simulink model "Demo_IntelligentTrafficSigns_cs"  (Simulink 7.6)
settings: Solver=FixedStepDiscrete  SolverMode=SingleTasking  StartTime=0.0  StopTime=66  FixedStep=1/10  RelTol=1e-3
model workspace (MAT data in the model file):
  mbox_table : struct (value not decoded)
PreLoadFcn:
  | check_NUTS_defined
  | 
  | assignin('base',['model_closed_' bdroot],false);
InitFcn:
  | PreScanExperimentInitFcn(bdroot)
StopFcn:
  | PreScanExperimentStopFcn(bdroot);
  | 
undefined symbols (not defined in the file or in its folder): DiscIntNeverNeededParam, magnitude, permission, R_curve, Results, Results_20090205_104801, spare_10, spare_4, spare_5, spare_6, spare_7, spare_8, spare_9, velocity_limit, x_ini_VRML, y_ini_VRML, z_ini_VRML
libraries: simulink_extras x8, PreScanSimulinkLibrary x7, simulink x5, PreScanUsefulBlocks x3
(part 1 of 10)
CloseFcn:
  | evalin('base',['clear model_closed_' bdroot]);
settings Model: Creator=PRESCAN  LastModifiedBy=buildbot
settings OptimizationCC: InitFltsAndDblsToZero=on
settings DebuggingCC: SaveWithParameterizedLinksMsg=none  SignalResolutionControl=TryResolveAllWithWarning  MaxConsecutiveZCsMsg=warning  MultiTaskDSMMsg=warning  MultiTaskCondExecSysMsg=none
settings SFSimCC: SimParseCustomCode=off
settings RTWCC: IncludeERTFirstTime=on
settings GRTTargetCC: TargetFcnLib=ansi_tfl_tmw.mat

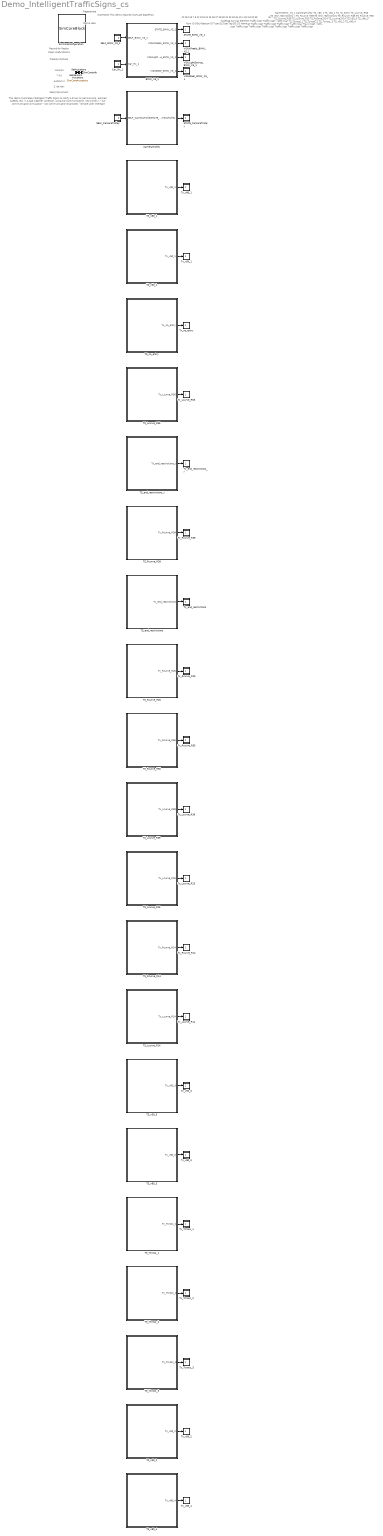
== system Demo_IntelligentTrafficSigns_cs ==
  note: OBU-Beacon ID
  note: Demo Help
  note: WARNING:
      | This demo requires MATLAB StateFlow
  note: Regenerate
  note: Record for Replay
  note: Open Useful Blocks
  note: PreScan Options
  note: Version:
  note: 7.4.0
  note: Author(s):
  note: S. de Hair
  note: Descriptive text:
  note: This demo illustrates Intelligent
      | Traffic Signs to notify a driver
      | on permissions, advised speeds,
      | etc. in a bad weather condition,
      | using C2I communication.
      |
      | Keyword(s):
      | - C2I communication simulation
      | - C2I communication examples
      | - Simple User Interface
  note: ID
      |
      | 28
      | 113
      | 7
      | 6
      | 22
      | 23
      | 8
      | 24
      | 25
      | 26
      | 27
      | 29
      | 30
      | 31
      | 32
      | 33
      | 34
      | 111
      | 112
      | 19
      | 20
      | 38
  note: OBU-Beacon ID
  note: TypeID
      |
      | 1
      | 5
      | 14
      | 14
      | 14
      | 14
      | 14
      | 14
      | 14
      | 14
      | 14
      | 14
      | 14
      | 14
      | 14
      | 14
      | 14
      | 14
      | 14
      | 14
      | 14
      | 14
  note: TypeTag
      |
      | Car
      | Cal element
      | Traffic sign
      | Traffic sign
      | Traffic sign
      | Traffic sign
      | Traffic sign
      | Traffic sign
      | Traffic sign
      | Traffic sign
      | Traffic sign
      | Traffic sign
      | Traffic sign
      | Traffic sign
      | Traffic sign
      | Traffic sign
      | Traffic sign
      | Traffic sign
      | Traffic sign
      | Traffic sign
      | Traffic sign
      | Traffic sign
  note: SD
      |
      | Y
      | Y
      | N
      | N
      | N
      | N
      | N
      | N
      | N
      | N
      | N
      | N
      | N
      | N
      | N
      | N
      | N
      | N
      | N
      | N
      | N
      | N
  note: CD
      |
      | N
      | N
      | N
      | N
      | N
      | N
      | N
      | N
      | N
      | N
      | N
      | N
      | N
      | N
      | N
      | N
      | N
      | N
      | N
      | N
      | N
      | N
  note: Name
      |
      | BMW_X5_1
      | CameraTrolley
      | TS_v80_1
      | TS_v50_1
      | TS_no_entry
      | TS_Lcurve_R55
      | TS_end_restrictions_r
      | TS_Rcurve_R39
      | TS_end_restrictions
      | TS_Rcurve_R25
      | TS_Rcurve_R55
      | TS_Lcurve_R39
      | TS_Lcurve_R25
      | TS_Rcurve_R14
      | TS_Lcurve_R14
      | TS_v50_3
      | TS_v80_3
      | TS_Tcross_1
      | TS_Tcross_2
      | TS_Tcross_3
      | TS_v50_2
      | TS_v80_4
  note: Num ID OBU-Beacon ID Type ID Type Tag SD CD Name
  Actuators : DataStoreMemory  Tag=Actuators  DataStoreName=SimCoreActuators  InitialValue=zeros(4358,1)
      settings: DeleteFcn=PreScanBlockDeleteFcn;
  BMW_X5_1 : Subsystem  Tag=WorldObjectSubSystem  InitFcn=UniqueId = '93';
      mask Display: image(imread('BMW_X5.jpg'));color('white');text(0,0,' ID=28\n')
  CameraTrolley : Subsystem  Tag=WorldObjectSubSystem  InitFcn=UniqueId = '98';
      mask Display: image(imread('Sphere.jpg'));color('white');text(0,0,' ID=113\n')
  Car_Rx_1 : Subsystem  Tag=AntennaR  Priority=3  mask(PreScan Source Block: Antenna Receiver){Channel=1, NrEntitiesOut=5, PortName=Car_Rx_1, PortIndex=8, PortWidth=1140, TimeStep=1/10}
      mask prompts: Channel "User Specified: Channel# [-]"; NrEntitiesOut "User Specified: # of Visible Actors"; PortName "Auto Assigned: Port Name [-]"; PortIndex "Auto Assigned: Index [-]"; PortWidth "Auto Assigned: Port Dimensions [-]"; TimeStep "Auto Assigned: Upload Period [s]"
      mask help:
      | Using the Antenna Receiver (RX) and Transmitter (TX) information between actors can be exchanged. This is done over a channel number to be specified by the user.
      |
      | The Antenna Reciever receives actor information and user-definable signals.
      |
      | For more information, please click Help.
      settings: DeleteFcn=PreScanBlockDeleteFcn;
      mask Help: eval('openHelp(424)')
      mask Display: image(imread('Rx.png'))
  GetSensors : Subsystem  Tag=GetSensors  Priority=2  mask(PreScan Simulation Core Block){NrOfSensors=1156, TimeStep=1/10}
      mask prompts: NrOfSensors "Number of sensor values"; TimeStep "Timestep [s]"
      mask help:
      | Please do not remove this block from the PreScan compilation sheet,
      | as it contains essential configuration parameters for a PreScan simulation.
      settings: DeleteFcn=PreScanBlockDeleteFcn;
  SELF_BMW_X5_1 : Subsystem  Tag=SELF  Priority=3  mask(PreScan Source Block: SELF){PortName=SELF_BMW_X5_1, PortIndex=0, PortWidth=8, TimeStep=1/10}
      mask prompts: PortName "Auto-Assigned: Port Name [-]"; PortIndex "Auto-Assigned: Port Index [-]"; PortWidth "Auto-Assigned: Port Dimensions"; TimeStep "Auto-Assigned: Upload Period [s]"
      mask help:
      | The SELF port shows (state) information of the actor itself. The outputs are position in the experiment's axis system and the GPS coordinates, and the object's absolute velocity and compass heading.
      |
      | For more information, please click Help.
      settings: DeleteFcn=PreScanBlockDeleteFcn;
      mask Help: eval('openHelp(462)')
      mask Display: image(imread('GPS.png'))
  SELF_CameraTrolley : Subsystem  Tag=SELF  Priority=3  mask(PreScan Source Block: SELF){PortName=SELF_CameraTrolley, PortIndex=1148, PortWidth=8, TimeStep=1/10}
      mask prompts: PortName "Auto-Assigned: Port Name [-]"; PortIndex "Auto-Assigned: Port Index [-]"; PortWidth "Auto-Assigned: Port Dimensions"; TimeStep "Auto-Assigned: Upload Period [s]"
      mask help:
      | The SELF port shows (state) information of the actor itself. The outputs are position in the experiment's axis system and the GPS coordinates, and the object's absolute velocity and compass heading.
      |
      | For more information, please click Help.
      settings: DeleteFcn=PreScanBlockDeleteFcn;
      mask Help: eval('openHelp(462)')
      mask Display: image(imread('GPS.png'))
  STATE_BMW_X5_1 : Subsystem  Tag=State  Priority=0  mask(PreScan Sink Block: State){x0=80, y0=1.75, z0=0.69, Roll0=0, Pitch0=0, Yaw0=179.999588, TimeStep=1/10}
      mask prompts: x0 "Ini x-position VEHICLE wrt GLOBAL axis system [m]"; y0 "Ini y-position VEHICLE wrt GLOBAL axis system [m]"; z0 "Ini z-position VEHICLE wrt GLOBAL axis system [m]"; Roll0 "Ini roll-angle BODY wrt VEHICLE axis system [deg]"; Pitch0 "Ini pitch-angle BODY wrt VEHICLE axis system [deg]"; Yaw0 "Ini yaw-angle VEHICLE wrt GLOBAL axis system [deg]"; TimeStep "Time Step [s]"
      mask help:
      | The Actor State contains a vector signal of size (9x1), containing the following signals:
      |
      | -  Position                  (x,y,z)                                     [m]
      | -  Velocity                  (vx,vy,vz)                               [m/s]
      | -  Angles                    (Roll, Pitch, Yaw)                   [rad]
      |
      | The Actor state is defined in the global axis system.
      | The state is superponed on the Initial position defined in the GUI.
      |
      | For more information, please click Help.
      settings: DeleteFcn=PreScanBlockDeleteFcn;
      mask Help: eval('openHelp(222)')
      mask Display: image(imread('State.png'))
  STATE_CameraTrolley : Subsystem  Tag=State  Priority=0  mask(PreScan Sink Block: State){x0=80, y0=1.75, z0=0.69, Roll0=0, Pitch0=0, Yaw0=179.9996, TimeStep=1/10}
      mask prompts: x0 "Ini x-position VEHICLE wrt GLOBAL axis system [m]"; y0 "Ini y-position VEHICLE wrt GLOBAL axis system [m]"; z0 "Ini z-position VEHICLE wrt GLOBAL axis system [m]"; Roll0 "Ini roll-angle BODY wrt VEHICLE axis system [deg]"; Pitch0 "Ini pitch-angle BODY wrt VEHICLE axis system [deg]"; Yaw0 "Ini yaw-angle VEHICLE wrt GLOBAL axis system [deg]"; TimeStep "Time Step [s]"
      mask help:
      | The Actor State contains a vector signal of size (9x1), containing the following signals:
      |
      | -  Position                  (x,y,z)                                     [m]
      | -  Velocity                  (vx,vy,vz)                               [m/s]
      | -  Angles                    (Roll, Pitch, Yaw)                   [rad]
      |
      | The Actor state is defined in the global axis system.
      | The state is superponed on the Initial position defined in the GUI.
      |
      | For more information, please click Help.
      settings: DeleteFcn=PreScanBlockDeleteFcn;
      mask Help: eval('openHelp(222)')
      mask Display: image(imread('State.png'))
  SetActuators : Subsystem  Tag=SetActuators  Priority=1  mask(PreScan Simulation Core Block){TimeStep=1/10, NrOfActuators=4358}
      mask prompts: TimeStep "Timestep [s]"; NrOfActuators "Number of actuator values"
      mask help:
      | Please do not remove this block from the PreScan compilation sheet,
      | as it contains essential configuration parameters for a PreScan simulation.
      settings: DeleteFcn=PreScanBlockDeleteFcn;
  SimCoreConfiguration : M-S-Function  Tag=SimCoreConfiguration  Description=8F06161321348DF6F304B7355CE55985CA9F2FDCA8A28FBBC2716AEAFE3B5E23  FunctionName=SimCoreBlock  Parameters=ExperimentXML PortsXML SimulationCore HostName CheckStart EnableLog RfR EnableGPUAccel ShowComputationTime  mask(PreScan Simulation Core Configurator){SimulationCore=NUTS, HostName=0, CheckStart=0, prescanversion='7.4.0', EnableLog=off, RfR=0, EnableGPUAccel=off, ShowComputationTime=off}
      mask prompts: SimulationCore "Simulation Core"; HostName "Simulation Host"; CheckStart "Show START Dialog"; prescanversion "PreScan Version"; EnableLog "Create TA Log"; RfR "Record for Replay"; EnableGPUAccel "Enable GPU Acceleration"; ShowComputationTime "Show ComputationTime Scope"
      mask help:
      | Please do not remove this block from the PreScan compilation sheet,
      | as it contains essential configuration parameter for a PreScan simulation.
      mask init:
      | model = bdroot;
      | ExperimentXML = [model(1:end-3) '.pex'];
      | PortsXML = [model '_ports.pcd'];
      settings: DeleteFcn=PreScanBlockDeleteFcn;  ModelCloseFcn=assignin('base',['model_closed_' bdroot],true);
      mask Help: eval('openHelp(812)')
      mask Display: image(imread('prescanlogo.ico'))
  SimCoreInfo : Subsystem  Tag=SimCoreInfo
  TS_Lcurve_R14 : Subsystem  Tag=WorldObjectSubSystem  InitFcn=UniqueId = '76';
      mask Display: image(imread('TrafficSignPoleNL.jpg'));color('white');text(0,0,' ID=32\n')
  TS_Lcurve_R25 : Subsystem  Tag=WorldObjectSubSystem  InitFcn=UniqueId = '72';
      mask Display: image(imread('TrafficSignPoleNL.jpg'));color('white');text(0,0,' ID=30\n')
  TS_Lcurve_R39 : Subsystem  Tag=WorldObjectSubSystem  InitFcn=UniqueId = '70';
      mask Display: image(imread('TrafficSignPoleNL.jpg'));color('white');text(0,0,' ID=29\n')
  TS_Lcurve_R55 : Subsystem  Tag=WorldObjectSubSystem  InitFcn=UniqueId = '58';
      mask Display: image(imread('TrafficSignPoleNL.jpg'));color('white');text(0,0,' ID=23\n')
  TS_Rcurve_R14 : Subsystem  Tag=WorldObjectSubSystem  InitFcn=UniqueId = '74';
      mask Display: image(imread('TrafficSignPoleNL.jpg'));color('white');text(0,0,' ID=31\n')
  TS_Rcurve_R25 : Subsystem  Tag=WorldObjectSubSystem  InitFcn=UniqueId = '66';
      mask Display: image(imread('TrafficSignPoleNL.jpg'));color('white');text(0,0,' ID=26\n')
  TS_Rcurve_R39 : Subsystem  Tag=WorldObjectSubSystem  InitFcn=UniqueId = '62';
      mask Display: image(imread('TrafficSignPoleNL.jpg'));color('white');text(0,0,' ID=24\n')
  TS_Rcurve_R55 : Subsystem  Tag=WorldObjectSubSystem  InitFcn=UniqueId = '68';
      mask Display: image(imread('TrafficSignPoleNL.jpg'));color('white');text(0,0,' ID=27\n')
  TS_Tcross_1 : Subsystem  Tag=WorldObjectSubSystem  InitFcn=UniqueId = '82';
      mask Display: image(imread('TrafficSignPoleNL.jpg'));color('white');text(0,0,' ID=111\n')
  TS_Tcross_2 : Subsystem  Tag=WorldObjectSubSystem  InitFcn=UniqueId = '84';
      mask Display: image(imread('TrafficSignPoleNL.jpg'));color('white');text(0,0,' ID=112\n')
  TS_Tcross_3 : Subsystem  Tag=WorldObjectSubSystem  InitFcn=UniqueId = '120';
      mask Display: image(imread('TrafficSignPoleNL.jpg'));color('white');text(0,0,' ID=19\n')
  TS_end_restrictions : Subsystem  Tag=WorldObjectSubSystem  InitFcn=UniqueId = '64';
      mask Display: image(imread('TrafficSignPoleNL.jpg'));color('white');text(0,0,' ID=25\n')
  TS_end_restrictions_r : Subsystem  Tag=WorldObjectSubSystem  InitFcn=UniqueId = '60';
      mask Display: image(imread('TrafficSignPoleNL.jpg'));color('white');text(0,0,' ID=8\n')
  TS_no_entry : Subsystem  Tag=WorldObjectSubSystem  InitFcn=UniqueId = '56';
      mask Display: image(imread('TrafficSignPoleNL.jpg'));color('white');text(0,0,' ID=22\n')
  TS_v50_1 : Subsystem  Tag=WorldObjectSubSystem  InitFcn=UniqueId = '54';
      mask Display: image(imread('TrafficSignPoleNL.jpg'));color('white');text(0,0,' ID=6\n')
  TS_v50_2 : Subsystem  Tag=WorldObjectSubSystem  InitFcn=UniqueId = '122';
      mask Display: image(imread('TrafficSignPoleNL.jpg'));color('white');text(0,0,' ID=20\n')
  TS_v50_3 : Subsystem  Tag=WorldObjectSubSystem  InitFcn=UniqueId = '78';
      mask Display: image(imread('TrafficSignPoleNL.jpg'));color('white');text(0,0,' ID=33\n')
  TS_v80_1 : Subsystem  Tag=WorldObjectSubSystem  InitFcn=UniqueId = '52';
      mask Display: image(imread('TrafficSignPoleNL.jpg'));color('white');text(0,0,' ID=7\n')
  TS_v80_3 : Subsystem  Tag=WorldObjectSubSystem  InitFcn=UniqueId = '80';
      mask Display: image(imread('TrafficSignPoleNL.jpg'));color('white');text(0,0,' ID=34\n')
  TS_v80_4 : Subsystem  Tag=WorldObjectSubSystem  InitFcn=UniqueId = '125';
      mask Display: image(imread('TrafficSignPoleNL.jpg'));color('white');text(0,0,' ID=38\n')
  Tx_Lcurve_R14 : Subsystem  Tag=AntennaT  Priority=0  mask(PreScan Sink Block: AntennaT){Channel=1, PortName=Tx_Lcurve_R14, PortIndex=2718, PortWidth=205, TimeStep=1/10}
      mask prompts: Channel "User Specified: Channel# [-]"; PortName "Auto Assigned: Port Name [-]"; PortIndex "Auto Assigned: Index [-]"; PortWidth "Auto Assigned: Port Dimensions [-]"; TimeStep "Auto Assigned: Upload Period [s]"
      mask help:
      | Using the Antenna Receiver (RX) and Transmitter (TX) information between actors can be exchanged. This is done over a channel number to be specified by the user.
      |
      | The Antenna Transmitter contains the following, user-definable signals:
      |
      | - Warning Signal ID	[-]
      | - Maneuvre ID		[-]
      | - Vehicle Type 		[-]
      | - Braking Capacity	[-]
      | - Vehicle Size		[-]
      | - Spares (1:10)		[-]
      |
      | For more information, please click Help.
      settings: DeleteFcn=PreScanBlockDeleteFcn;
      mask Help: eval('openHelp(424)')
      mask Display: image(imread('Tx.png'))
  Tx_Lcurve_R25 : Subsystem  Tag=AntennaT  Priority=0  mask(PreScan Sink Block: AntennaT){Channel=1, PortName=Tx_Lcurve_R25, PortIndex=2308, PortWidth=205, TimeStep=1/10}
      mask prompts: Channel "User Specified: Channel# [-]"; PortName "Auto Assigned: Port Name [-]"; PortIndex "Auto Assigned: Index [-]"; PortWidth "Auto Assigned: Port Dimensions [-]"; TimeStep "Auto Assigned: Upload Period [s]"
      mask help:
      | Using the Antenna Receiver (RX) and Transmitter (TX) information between actors can be exchanged. This is done over a channel number to be specified by the user.
      |
      | The Antenna Transmitter contains the following, user-definable signals:
      |
      | - Warning Signal ID	[-]
      | - Maneuvre ID		[-]
      | - Vehicle Type 		[-]
      | - Braking Capacity	[-]
      | - Vehicle Size		[-]
      | - Spares (1:10)		[-]
      |
      | For more information, please click Help.
      settings: DeleteFcn=PreScanBlockDeleteFcn;
      mask Help: eval('openHelp(424)')
      mask Display: image(imread('Tx.png'))
  Tx_Lcurve_R39 : Subsystem  Tag=AntennaT  Priority=0  mask(PreScan Sink Block: AntennaT){Channel=1, PortName=Tx_Lcurve_R39, PortIndex=2103, PortWidth=205, TimeStep=1/10}
      mask prompts: Channel "User Specified: Channel# [-]"; PortName "Auto Assigned: Port Name [-]"; PortIndex "Auto Assigned: Index [-]"; PortWidth "Auto Assigned: Port Dimensions [-]"; TimeStep "Auto Assigned: Upload Period [s]"
      mask help:
      | Using the Antenna Receiver (RX) and Transmitter (TX) information between actors can be exchanged. This is done over a channel number to be specified by the user.
      |
      | The Antenna Transmitter contains the following, user-definable signals:
      |
      | - Warning Signal ID	[-]
      | - Maneuvre ID		[-]
      | - Vehicle Type 		[-]
      | - Braking Capacity	[-]
      | - Vehicle Size		[-]
      | - Spares (1:10)		[-]
      |
      | For more information, please click Help.
      settings: DeleteFcn=PreScanBlockDeleteFcn;
      mask Help: eval('openHelp(424)')
      mask Display: image(imread('Tx.png'))
  Tx_Lcurve_R55 : Subsystem  Tag=AntennaT  Priority=0  mask(PreScan Sink Block: AntennaT){Channel=1, PortName=Tx_Lcurve_R55, PortIndex=873, PortWidth=205, TimeStep=1/10}
      mask prompts: Channel "User Specified: Channel# [-]"; PortName "Auto Assigned: Port Name [-]"; PortIndex "Auto Assigned: Index [-]"; PortWidth "Auto Assigned: Port Dimensions [-]"; TimeStep "Auto Assigned: Upload Period [s]"
      mask help:
      | Using the Antenna Receiver (RX) and Transmitter (TX) information between actors can be exchanged. This is done over a channel number to be specified by the user.
      |
      | The Antenna Transmitter contains the following, user-definable signals:
      |
      | - Warning Signal ID	[-]
      | - Maneuvre ID		[-]
      | - Vehicle Type 		[-]
      | - Braking Capacity	[-]
      | - Vehicle Size		[-]
      | - Spares (1:10)		[-]
      |
      | For more information, please click Help.
      settings: DeleteFcn=PreScanBlockDeleteFcn;
      mask Help: eval('openHelp(424)')
      mask Display: image(imread('Tx.png'))
  Tx_Rcurve_R14 : Subsystem  Tag=AntennaT  Priority=0  mask(PreScan Sink Block: AntennaT){Channel=1, PortName=Tx_Rcurve_R14, PortIndex=2513, PortWidth=205, TimeStep=1/10}
      mask prompts: Channel "User Specified: Channel# [-]"; PortName "Auto Assigned: Port Name [-]"; PortIndex "Auto Assigned: Index [-]"; PortWidth "Auto Assigned: Port Dimensions [-]"; TimeStep "Auto Assigned: Upload Period [s]"
      mask help:
      | Using the Antenna Receiver (RX) and Transmitter (TX) information between actors can be exchanged. This is done over a channel number to be specified by the user.
      |
      | The Antenna Transmitter contains the following, user-definable signals:
      |
      | - Warning Signal ID	[-]
      | - Maneuvre ID		[-]
      | - Vehicle Type 		[-]
      | - Braking Capacity	[-]
      | - Vehicle Size		[-]
      | - Spares (1:10)		[-]
      |
      | For more information, please click Help.
      settings: DeleteFcn=PreScanBlockDeleteFcn;
      mask Help: eval('openHelp(424)')
      mask Display: image(imread('Tx.png'))
  Tx_Rcurve_R25 : Subsystem  Tag=AntennaT  Priority=0  mask(PreScan Sink Block: AntennaT){Channel=1, PortName=Tx_Rcurve_R25, PortIndex=1693, PortWidth=205, TimeStep=1/10}
      mask prompts: Channel "User Specified: Channel# [-]"; PortName "Auto Assigned: Port Name [-]"; PortIndex "Auto Assigned: Index [-]"; PortWidth "Auto Assigned: Port Dimensions [-]"; TimeStep "Auto Assigned: Upload Period [s]"
      mask help:
      | Using the Antenna Receiver (RX) and Transmitter (TX) information between actors can be exchanged. This is done over a channel number to be specified by the user.
      |
      | The Antenna Transmitter contains the following, user-definable signals:
      |
      | - Warning Signal ID	[-]
      | - Maneuvre ID		[-]
      | - Vehicle Type 		[-]
      | - Braking Capacity	[-]
      | - Vehicle Size		[-]
      | - Spares (1:10)		[-]
      |
      | For more information, please click Help.
      settings: DeleteFcn=PreScanBlockDeleteFcn;
      mask Help: eval('openHelp(424)')
      mask Display: image(imread('Tx.png'))
  Tx_Rcurve_R39 : Subsystem  Tag=AntennaT  Priority=0  mask(PreScan Sink Block: AntennaT){Channel=1, PortName=Tx_Rcurve_R39, PortIndex=1283, PortWidth=205, TimeStep=1/10}
      mask prompts: Channel "User Specified: Channel# [-]"; PortName "Auto Assigned: Port Name [-]"; PortIndex "Auto Assigned: Index [-]"; PortWidth "Auto Assigned: Port Dimensions [-]"; TimeStep "Auto Assigned: Upload Period [s]"
      mask help:
      | Using the Antenna Receiver (RX) and Transmitter (TX) information between actors can be exchanged. This is done over a channel number to be specified by the user.
      |
      | The Antenna Transmitter contains the following, user-definable signals:
      |
      | - Warning Signal ID	[-]
      | - Maneuvre ID		[-]
      | - Vehicle Type 		[-]
      | - Braking Capacity	[-]
      | - Vehicle Size		[-]
      | - Spares (1:10)		[-]
      |
      | For more information, please click Help.
      settings: DeleteFcn=PreScanBlockDeleteFcn;
      mask Help: eval('openHelp(424)')
      mask Display: image(imread('Tx.png'))
  Tx_Rcurve_R55 : Subsystem  Tag=AntennaT  Priority=0  mask(PreScan Sink Block: AntennaT){Channel=1, PortName=Tx_Rcurve_R55, PortIndex=1898, PortWidth=205, TimeStep=1/10}
      mask prompts: Channel "User Specified: Channel# [-]"; PortName "Auto Assigned: Port Name [-]"; PortIndex "Auto Assigned: Index [-]"; PortWidth "Auto Assigned: Port Dimensions [-]"; TimeStep "Auto Assigned: Upload Period [s]"
      mask help:
      | Using the Antenna Receiver (RX) and Transmitter (TX) information between actors can be exchanged. This is done over a channel number to be specified by the user.
      |
      | The Antenna Transmitter contains the following, user-definable signals:
      |
      | - Warning Signal ID	[-]
      | - Maneuvre ID		[-]
      | - Vehicle Type 		[-]
      | - Braking Capacity	[-]
      | - Vehicle Size		[-]
      | - Spares (1:10)		[-]
      |
      | For more information, please click Help.
      settings: DeleteFcn=PreScanBlockDeleteFcn;
      mask Help: eval('openHelp(424)')
      mask Display: image(imread('Tx.png'))
  Tx_Tcross_1 : Subsystem  Tag=AntennaT  Priority=0  mask(PreScan Sink Block: AntennaT){Channel=1, PortName=Tx_Tcross_1, PortIndex=3333, PortWidth=205, TimeStep=1/10}
      mask prompts: Channel "User Specified: Channel# [-]"; PortName "Auto Assigned: Port Name [-]"; PortIndex "Auto Assigned: Index [-]"; PortWidth "Auto Assigned: Port Dimensions [-]"; TimeStep "Auto Assigned: Upload Period [s]"
      mask help:
      | Using the Antenna Receiver (RX) and Transmitter (TX) information between actors can be exchanged. This is done over a channel number to be specified by the user.
      |
      | The Antenna Transmitter contains the following, user-definable signals:
      |
      | - Warning Signal ID	[-]
      | - Maneuvre ID		[-]
      | - Vehicle Type 		[-]
      | - Braking Capacity	[-]
      | - Vehicle Size		[-]
      | - Spares (1:10)		[-]
      |
      | For more information, please click Help.
      settings: DeleteFcn=PreScanBlockDeleteFcn;
      mask Help: eval('openHelp(424)')
      mask Display: image(imread('Tx.png'))
  Tx_Tcross_2 : Subsystem  Tag=AntennaT  Priority=0  mask(PreScan Sink Block: AntennaT){Channel=1, PortName=Tx_Tcross_2, PortIndex=3538, PortWidth=205, TimeStep=1/10}
      mask prompts: Channel "User Specified: Channel# [-]"; PortName "Auto Assigned: Port Name [-]"; PortIndex "Auto Assigned: Index [-]"; PortWidth "Auto Assigned: Port Dimensions [-]"; TimeStep "Auto Assigned: Upload Period [s]"
      mask help:
      | Using the Antenna Receiver (RX) and Transmitter (TX) information between actors can be exchanged. This is done over a channel number to be specified by the user.
      |
      | The Antenna Transmitter contains the following, user-definable signals:
      |
      | - Warning Signal ID	[-]
      | - Maneuvre ID		[-]
      | - Vehicle Type 		[-]
      | - Braking Capacity	[-]
      | - Vehicle Size		[-]
      | - Spares (1:10)		[-]
      |
      | For more information, please click Help.
      settings: DeleteFcn=PreScanBlockDeleteFcn;
      mask Help: eval('openHelp(424)')
      mask Display: image(imread('Tx.png'))
  Tx_Tcross_3 : Subsystem  Tag=AntennaT  Priority=0  mask(PreScan Sink Block: AntennaT){Channel=1, PortName=Tx_Tcross_3, PortIndex=3743, PortWidth=205, TimeStep=1/10}
      mask prompts: Channel "User Specified: Channel# [-]"; PortName "Auto Assigned: Port Name [-]"; PortIndex "Auto Assigned: Index [-]"; PortWidth "Auto Assigned: Port Dimensions [-]"; TimeStep "Auto Assigned: Upload Period [s]"
      mask help:
      | Using the Antenna Receiver (RX) and Transmitter (TX) information between actors can be exchanged. This is done over a channel number to be specified by the user.
      |
      | The Antenna Transmitter contains the following, user-definable signals:
      |
      | - Warning Signal ID	[-]
      | - Maneuvre ID		[-]
      | - Vehicle Type 		[-]
      | - Braking Capacity	[-]
      | - Vehicle Size		[-]
      | - Spares (1:10)		[-]
      |
      | For more information, please click Help.
      settings: DeleteFcn=PreScanBlockDeleteFcn;
      mask Help: eval('openHelp(424)')
      mask Display: image(imread('Tx.png'))
  Tx_end_restrictions : Subsystem  Tag=AntennaT  Priority=0  mask(PreScan Sink Block: AntennaT){Channel=1, PortName=Tx_end_restrictions, PortIndex=1488, PortWidth=205, TimeStep=1/10}
      mask prompts: Channel "User Specified: Channel# [-]"; PortName "Auto Assigned: Port Name [-]"; PortIndex "Auto Assigned: Index [-]"; PortWidth "Auto Assigned: Port Dimensions [-]"; TimeStep "Auto Assigned: Upload Period [s]"
      mask help:
      | Using the Antenna Receiver (RX) and Transmitter (TX) information between actors can be exchanged. This is done over a channel number to be specified by the user.
      |
      | The Antenna Transmitter contains the following, user-definable signals:
      |
      | - Warning Signal ID	[-]
      | - Maneuvre ID		[-]
      | - Vehicle Type 		[-]
      | - Braking Capacity	[-]
      | - Vehicle Size		[-]
      | - Spares (1:10)		[-]
      |
      | For more information, please click Help.
      settings: DeleteFcn=PreScanBlockDeleteFcn;
      mask Help: eval('openHelp(424)')
      mask Display: image(imread('Tx.png'))
  Tx_end_restrictions_r : Subsystem  Tag=AntennaT  Priority=0  mask(PreScan Sink Block: AntennaT){Channel=1, PortName=Tx_end_restrictions_r, PortIndex=1078, PortWidth=205, TimeStep=1/10}
      mask prompts: Channel "User Specified: Channel# [-]"; PortName "Auto Assigned: Port Name [-]"; PortIndex "Auto Assigned: Index [-]"; PortWidth "Auto Assigned: Port Dimensions [-]"; TimeStep "Auto Assigned: Upload Period [s]"
      mask help:
      | Using the Antenna Receiver (RX) and Transmitter (TX) information between actors can be exchanged. This is done over a channel number to be specified by the user.
      |
      | The Antenna Transmitter contains the following, user-definable signals:
      |
      | - Warning Signal ID	[-]
      | - Maneuvre ID		[-]
      | - Vehicle Type 		[-]
      | - Braking Capacity	[-]
      | - Vehicle Size		[-]
      | - Spares (1:10)		[-]
      |
      | For more information, please click Help.
      settings: DeleteFcn=PreScanBlockDeleteFcn;
      mask Help: eval('openHelp(424)')
      mask Display: image(imread('Tx.png'))
  Tx_no_entry : Subsystem  Tag=AntennaT  Priority=0  mask(PreScan Sink Block: AntennaT){Channel=1, PortName=Tx_no_entry, PortIndex=668, PortWidth=205, TimeStep=1/10}
      mask prompts: Channel "User Specified: Channel# [-]"; PortName "Auto Assigned: Port Name [-]"; PortIndex "Auto Assigned: Index [-]"; PortWidth "Auto Assigned: Port Dimensions [-]"; TimeStep "Auto Assigned: Upload Period [s]"
      mask help:
      | Using the Antenna Receiver (RX) and Transmitter (TX) information between actors can be exchanged. This is done over a channel number to be specified by the user.
      |
      | The Antenna Transmitter contains the following, user-definable signals:
      |
      | - Warning Signal ID	[-]
      | - Maneuvre ID		[-]
      | - Vehicle Type 		[-]
      | - Braking Capacity	[-]
      | - Vehicle Size		[-]
      | - Spares (1:10)		[-]
      |
      | For more information, please click Help.
      settings: DeleteFcn=PreScanBlockDeleteFcn;
      mask Help: eval('openHelp(424)')
      mask Display: image(imread('Tx.png'))
  Tx_v50_1 : Subsystem  Tag=AntennaT  Priority=0  mask(PreScan Sink Block: AntennaT){Channel=1, PortName=Tx_v50_1, PortIndex=463, PortWidth=205, TimeStep=1/10}
      mask prompts: Channel "User Specified: Channel# [-]"; PortName "Auto Assigned: Port Name [-]"; PortIndex "Auto Assigned: Index [-]"; PortWidth "Auto Assigned: Port Dimensions [-]"; TimeStep "Auto Assigned: Upload Period [s]"
      mask help:
      | Using the Antenna Receiver (RX) and Transmitter (TX) information between actors can be exchanged. This is done over a channel number to be specified by the user.
      |
      | The Antenna Transmitter contains the following, user-definable signals:
      |
      | - Warning Signal ID	[-]
      | - Maneuvre ID		[-]
      | - Vehicle Type 		[-]
      | - Braking Capacity	[-]
      | - Vehicle Size		[-]
      | - Spares (1:10)		[-]
      |
      | For more information, please click Help.
      settings: DeleteFcn=PreScanBlockDeleteFcn;
      mask Help: eval('openHelp(424)')
      mask Display: image(imread('Tx.png'))
  Tx_v50_2 : Subsystem  Tag=AntennaT  Priority=0  mask(PreScan Sink Block: AntennaT){Channel=1, PortName=Tx_v50_2, PortIndex=3948, PortWidth=205, TimeStep=1/10}
      mask prompts: Channel "User Specified: Channel# [-]"; PortName "Auto Assigned: Port Name [-]"; PortIndex "Auto Assigned: Index [-]"; PortWidth "Auto Assigned: Port Dimensions [-]"; TimeStep "Auto Assigned: Upload Period [s]"
      mask help:
      | Using the Antenna Receiver (RX) and Transmitter (TX) information between actors can be exchanged. This is done over a channel number to be specified by the user.
      |
      | The Antenna Transmitter contains the following, user-definable signals:
      |
      | - Warning Signal ID	[-]
      | - Maneuvre ID		[-]
      | - Vehicle Type 		[-]
      | - Braking Capacity	[-]
      | - Vehicle Size		[-]
      | - Spares (1:10)		[-]
      |
      | For more information, please click Help.
      settings: DeleteFcn=PreScanBlockDeleteFcn;
      mask Help: eval('openHelp(424)')
      mask Display: image(imread('Tx.png'))
  Tx_v50_3 : Subsystem  Tag=AntennaT  Priority=0  mask(PreScan Sink Block: AntennaT){Channel=1, PortName=Tx_v50_3, PortIndex=2923, PortWidth=205, TimeStep=1/10}
      mask prompts: Channel "User Specified: Channel# [-]"; PortName "Auto Assigned: Port Name [-]"; PortIndex "Auto Assigned: Index [-]"; PortWidth "Auto Assigned: Port Dimensions [-]"; TimeStep "Auto Assigned: Upload Period [s]"
      mask help:
      | Using the Antenna Receiver (RX) and Transmitter (TX) information between actors can be exchanged. This is done over a channel number to be specified by the user.
      |
      | The Antenna Transmitter contains the following, user-definable signals:
      |
      | - Warning Signal ID	[-]
      | - Maneuvre ID		[-]
      | - Vehicle Type 		[-]
      | - Braking Capacity	[-]
      | - Vehicle Size		[-]
      | - Spares (1:10)		[-]
      |
      | For more information, please click Help.
      settings: DeleteFcn=PreScanBlockDeleteFcn;
      mask Help: eval('openHelp(424)')
      mask Display: image(imread('Tx.png'))
  Tx_v80_1 : Subsystem  Tag=AntennaT  Priority=0  mask(PreScan Sink Block: AntennaT){Channel=1, PortName=Tx_v80_1, PortIndex=258, PortWidth=205, TimeStep=1/10}
      mask prompts: Channel "User Specified: Channel# [-]"; PortName "Auto Assigned: Port Name [-]"; PortIndex "Auto Assigned: Index [-]"; PortWidth "Auto Assigned: Port Dimensions [-]"; TimeStep "Auto Assigned: Upload Period [s]"
      mask help:
      | Using the Antenna Receiver (RX) and Transmitter (TX) information between actors can be exchanged. This is done over a channel number to be specified by the user.
      |
      | The Antenna Transmitter contains the following, user-definable signals:
      |
      | - Warning Signal ID	[-]
      | - Maneuvre ID		[-]
      | - Vehicle Type 		[-]
      | - Braking Capacity	[-]
      | - Vehicle Size		[-]
      | - Spares (1:10)		[-]
      |
      | For more information, please click Help.
      settings: DeleteFcn=PreScanBlockDeleteFcn;
      mask Help: eval('openHelp(424)')
      mask Display: image(imread('Tx.png'))
  Tx_v80_3 : Subsystem  Tag=AntennaT  Priority=0  mask(PreScan Sink Block: AntennaT){Channel=1, PortName=Tx_v80_3, PortIndex=3128, PortWidth=205, TimeStep=1/10}
      mask prompts: Channel "User Specified: Channel# [-]"; PortName "Auto Assigned: Port Name [-]"; PortIndex "Auto Assigned: Index [-]"; PortWidth "Auto Assigned: Port Dimensions [-]"; TimeStep "Auto Assigned: Upload Period [s]"
      mask help:
      | Using the Antenna Receiver (RX) and Transmitter (TX) information between actors can be exchanged. This is done over a channel number to be specified by the user.
      |
      | The Antenna Transmitter contains the following, user-definable signals:
      |
      | - Warning Signal ID	[-]
      | - Maneuvre ID		[-]
      | - Vehicle Type 		[-]
      | - Braking Capacity	[-]
      | - Vehicle Size		[-]
      | - Spares (1:10)		[-]
      |
      | For more information, please click Help.
      settings: DeleteFcn=PreScanBlockDeleteFcn;
      mask Help: eval('openHelp(424)')
      mask Display: image(imread('Tx.png'))
  Tx_v80_4 : Subsystem  Tag=AntennaT  Priority=0  mask(PreScan Sink Block: AntennaT){Channel=1, PortName=Tx_v80_4, PortIndex=4153, PortWidth=205, TimeStep=1/10}
      mask prompts: Channel "User Specified: Channel# [-]"; PortName "Auto Assigned: Port Name [-]"; PortIndex "Auto Assigned: Index [-]"; PortWidth "Auto Assigned: Port Dimensions [-]"; TimeStep "Auto Assigned: Upload Period [s]"
      mask help:
      | Using the Antenna Receiver (RX) and Transmitter (TX) information between actors can be exchanged. This is done over a channel number to be specified by the user.
      |
      | The Antenna Transmitter contains the following, user-definable signals:
      |
      | - Warning Signal ID	[-]
      | - Maneuvre ID		[-]
      | - Vehicle Type 		[-]
      | - Braking Capacity	[-]
      | - Vehicle Size		[-]
      | - Spares (1:10)		[-]
      |
      | For more information, please click Help.
      settings: DeleteFcn=PreScanBlockDeleteFcn;
      mask Help: eval('openHelp(424)')
      mask Display: image(imread('Tx.png'))
  VISULightSource_BMW_X5_1 : Subsystem  Tag=VisuLightSource  Priority=0
      settings: DeleteFcn=PreScanBlockDeleteFcn;
      mask Type: PreScan Sink Block: VisuLightSource
      mask Description:
      | The VisuLightSource block provides a way to animate Light Sources of the indicator, braking, fog and main lights of a vehicle.
      | 
      | For more information, please click Help.
      mask Help: eval('openHelp(223)')
      mask Display: image(imread('RearLight.png'))
  VISUSteer_BMW_X5_1 : Subsystem  Tag=VisuSteer  Priority=0
      settings: DeleteFcn=PreScanBlockDeleteFcn;
      mask Type: PreScan Sink Block: VisuSteer
      mask Description:
      | The VisuSteer block provides a way to adapt the orientation of the steering column and steering wheel of a vehicle.
      | 
      | For more information, please click Help.
      mask Help: eval('openHelp(223)')
      mask Display: image(imread('steeringwheel.png'))
  VISUWheels_BMW_X5_1 : Subsystem  Tag=VisuWheels  Priority=0
      settings: DeleteFcn=PreScanBlockDeleteFcn;
      mask Type: PreScan Sink Block: VisuWheels
      mask Description:
      | The VisuWheels block provides a way to animate the wheels of a vehicle, with steering, rotation (because of velocity) and z-displacement.
      | 
      | For more information, please click Help.
      mask Help: eval('openHelp(223)')
      mask Display: image(imread('wheel.png'))
  wires:
  BMW_X5_1/1(STATE_BMW_X5_1) -> STATE_BMW_X5_1/1(State)
  SELF_BMW_X5_1/1(U) -> BMW_X5_1/1(SELF_BMW_X5_1)
  Car_Rx_1/1(U) -> BMW_X5_1/2(Car_Rx_1)
  BMW_X5_1/2(VISUWheels_BMW_X5_1) -> VISUWheels_BMW_X5_1/1(Wheels)
  BMW_X5_1/3(VISULightSource_BMW_X5_1) -> VISULightSource_BMW_X5_1/1(Lights)
  BMW_X5_1/4(VISUSteer_BMW_X5_1) -> VISUSteer_BMW_X5_1/1(Steer)
  CameraTrolley/1(STATE_CameraTrolley) -> STATE_CameraTrolley/1(State)
  SELF_CameraTrolley/1(U) -> CameraTrolley/1(SELF_CameraTrolley)
  TS_v80_1/1(Tx_v80_1) -> Tx_v80_1/1(Y)
  TS_v50_1/1(Tx_v50_1) -> Tx_v50_1/1(Y)
  TS_no_entry/1(Tx_no_entry) -> Tx_no_entry/1(Y)
  TS_Lcurve_R55/1(Tx_Lcurve_R55) -> Tx_Lcurve_R55/1(Y)
  TS_end_restrictions_r/1(Tx_end_restrictions_r) -> Tx_end_restrictions_r/1(Y)
  TS_Rcurve_R39/1(Tx_Rcurve_R39) -> Tx_Rcurve_R39/1(Y)
  TS_end_restrictions/1(Tx_end_restrictions) -> Tx_end_restrictions/1(Y)
  TS_Rcurve_R25/1(Tx_Rcurve_R25) -> Tx_Rcurve_R25/1(Y)
  TS_Rcurve_R55/1(Tx_Rcurve_R55) -> Tx_Rcurve_R55/1(Y)
  TS_Lcurve_R39/1(Tx_Lcurve_R39) -> Tx_Lcurve_R39/1(Y)
  TS_Lcurve_R25/1(Tx_Lcurve_R25) -> Tx_Lcurve_R25/1(Y)
  TS_Rcurve_R14/1(Tx_Rcurve_R14) -> Tx_Rcurve_R14/1(Y)
  TS_Lcurve_R14/1(Tx_Lcurve_R14) -> Tx_Lcurve_R14/1(Y)
  TS_v50_3/1(Tx_v50_3) -> Tx_v50_3/1(Y)
  TS_v80_3/1(Tx_v80_3) -> Tx_v80_3/1(Y)
  TS_Tcross_1/1(Tx_Tcross_1) -> Tx_Tcross_1/1(Y)
  TS_Tcross_2/1(Tx_Tcross_2) -> Tx_Tcross_2/1(Y)
  TS_Tcross_3/1(Tx_Tcross_3) -> Tx_Tcross_3/1(Y)
  TS_v50_2/1(Tx_v50_2) -> Tx_v50_2/1(Y)
  TS_v80_4/1(Tx_v80_4) -> Tx_v80_4/1(Y)
  SetActuators/1(dummy) -> GetSensors/1(dummy)

== system Demo_IntelligentTrafficSigns_cs/BMW_X5_1 ==
  SELF_BMW_X5_1 : Inport  Tag=SELFInport
  Car_Rx_1 : Inport  Tag=AntennaRInport  Port=2
  ANIMATION PORTS Tuning : Subsystem
  Car_Rx_1_BusSelector : BusSelector  Tag=BusAntennaR  OutputSignals=velocity_limit,permission,R_curve
  Car_Rx_1_Demux : Subsystem  Tag=DemuxAntennaR  mask(PreScan Demux Block:){Channel=1}
      settings: DeleteFcn=PreScanBlockDeleteFcn;
      mask Display:
      | fprintf('Channel: %d',Channel)
      | port_label('output', 1, 'Vehicle_ID')
      | port_label('output', 2, 'GPS Pos Lat [deg]')
      | port_label('output', 3, 'GPS Pos Long [deg]')
      | port_label('output', 4, 'GPS Pos Alt [m]')
      | port_label('output', 5, 'Velocity [m/s]')
      | port_label('output', 6, 'Acceleration [m/s2]')
      | port_label('output', 7, 'Heading [deg]')
      | port_label('output', 8, 'Warning signal ID')
      | port_label('output', 9, 'Maneuvre ID')
      | port_label('output', 10, 'Vehicle Type')
      | port_label('output', 11, 'Braking Capacity')
      | port_label('output', 12, 'Vehicle Size')
      | port_label('output', 13, 'Spare')
  Ground_{1..10,13} (11 alike) : Ground
  INTERPRETATION ALGORITHM TrafficSign Intelligence : Subsystem
      mask Display: image(imread('Pictures\TS_detection_algorithm.jpg'))
  SELF_Demux : Subsystem  Tag=DemuxSELF
      settings: DeleteFcn=PreScanBlockDeleteFcn;
      mask Type: PreScan Demux Block:
      mask Display:
      | port_label('output',1,'x [m]')
      | port_label('output',2,'y [m]')
      | port_label('output',3,'z [m]','texmode','on')
      | port_label('output',4,'Rotx [deg]','texmode','on')
      | port_label('output',5,'Roty [deg]','texmode','on')
      | port_label('output',6,'Rotz [deg]','texmode','on')
      | port_label('output',7,'GPS lat [deg]','texmode','on')
      | port_label('output',8,'GPS long [deg]','texmode','on')
      | port_label('output',9,'GPS alt [m]','texmode','on')
      | port_label('output',10,'Velocity [m/s]')
      | port_label('output',11,'Heading [deg]')
      | port_label('output',12,'Yaw rate [deg/s]')
  Terminator_{1..15} (15 alike) : Terminator
  Trajectories : Subsystem  Tag=Trajectories
      mask Display: image(imread('Trajectory.png'));
  VISULightSource_Mux : Subsystem  Tag=MuxLightSource
      settings: DeleteFcn=PreScanBlockDeleteFcn;
      mask Type: PreScan Mux Block:
  VISUSteer_Mux : Subsystem  Tag=MuxSteer
      settings: DeleteFcn=PreScanBlockDeleteFcn;
      mask Type: PreScan Mux Block:
  VISUWheels_Mux : Subsystem  Tag=MuxWheels  mask(PreScan Mux Block:){TimeStep=1/10}
      mask prompts: TimeStep "Auto Assigned: Time Step [s]"
      settings: DeleteFcn=PreScanBlockDeleteFcn;
  STATE_BMW_X5_1 : Outport  Tag=StateOutport
  VISUWheels_BMW_X5_1 : Outport  Tag=VisuWheelsOutport  Port=2  SampleTime=1/10
  VISULightSource_BMW_X5_1 : Outport  Tag=VisuLightsOutport  Port=3  SampleTime=1/10
  VISUSteer_BMW_X5_1 : Outport  Tag=VisuSteerOutport  Port=4  SampleTime=1/10
  tag scope: State_BMW=global
  wires:
  INTERPRETATION ALGORITHM TrafficSign Intelligence/1(delta_v [m/s]) -> [delta_v]
  [State_BMW] -> ANIMATION PORTS Tuning/2(state)
  [delta_v] -> ANIMATION PORTS Tuning/1(v_corr)
  SELF_BMW_X5_1/1 -> SELF_Demux/1(SELF)
  Car_Rx_1_Demux/13(spare) -> Car_Rx_1_BusSelector/1
  Car_Rx_1/1 -> Car_Rx_1_Demux/1(AntennaR)
  VISUWheels_Mux/1(Wheels) -> VISUWheels_BMW_X5_1/1
  VISULightSource_Mux/1(Lights) -> VISULightSource_BMW_X5_1/1
  VISUSteer_Mux/1(Steer) -> VISUSteer_BMW_X5_1/1
  Trajectories/1(State) -> STATE_BMW_X5_1/1, [State_BMW]
  ANIMATION PORTS Tuning/7(Brake [-]) -> VISULightSource_Mux/11(BrakeLightLeft), VISULightSource_Mux/12(BrakeLightRight)
  Ground_13/1 -> VISULightSource_Mux/3(FogLightRearLeft)
  [delta_v] -> Trajectories/1(v_corr [m/s])
  Car_Rx_1_BusSelector/1 -> INTERPRETATION ALGORITHM TrafficSign Intelligence/10(velocity_limits [km/h])  "<velocity_limit>"
  ANIMATION PORTS Tuning/6(zDisp RR [m]) -> VISUWheels_Mux/7(zDisp RR [m])
  ANIMATION PORTS Tuning/4(zDisp FR [m]) -> VISUWheels_Mux/5(zDisp FR [m])
  ANIMATION PORTS Tuning/2(Velocity [m/s]) -> VISUWheels_Mux/3(Velocity [m/s])
  ANIMATION PORTS Tuning/5(zDisp RL [m]) -> VISUWheels_Mux/6(zDisp RL [m])
  ANIMATION PORTS Tuning/3(zDisp FL [m]) -> VISUWheels_Mux/4(zDisp FL [m])
  ANIMATION PORTS Tuning/1(Steer Angle Front [deg]) -> VISUWheels_Mux/1(Steer Angle Front [deg])
  ANIMATION PORTS Tuning/9(Steer [deg]) -> VISUSteer_Mux/2(Steer [deg])
  ANIMATION PORTS Tuning/8(Column [deg]) -> VISUSteer_Mux/1(Column [deg])
  Car_Rx_1_Demux/3(GPS_pos_long_[deg]) -> INTERPRETATION ALGORITHM TrafficSign Intelligence/7(longs [deg])
  Car_Rx_1_Demux/2(GPS_pos_lat_[deg]) -> INTERPRETATION ALGORITHM TrafficSign Intelligence/6(lats [deg])
  Car_Rx_1_Demux/1(Vehicle_ID) -> INTERPRETATION ALGORITHM TrafficSign Intelligence/5(ids [-])
  SELF_Demux/11(heading [deg]) -> INTERPRETATION ALGORITHM TrafficSign Intelligence/4(heading-own [deg])
  SELF_Demux/10(velocity [m/s]) -> INTERPRETATION ALGORITHM TrafficSign Intelligence/3(v_host [m/s])
  SELF_Demux/2(y [m]) -> INTERPRETATION ALGORITHM TrafficSign Intelligence/2(y_host [m])
  SELF_Demux/1(x [m]) -> INTERPRETATION ALGORITHM TrafficSign Intelligence/1(x_host [m])
  Car_Rx_1_Demux/4(GPS_pos_alt_[m]) -> INTERPRETATION ALGORITHM TrafficSign Intelligence/8(alts [m])
  Car_Rx_1_Demux/7(Heading [deg]) -> INTERPRETATION ALGORITHM TrafficSign Intelligence/9(headings [deg])
  Car_Rx_1_BusSelector/3 -> INTERPRETATION ALGORITHM TrafficSign Intelligence/12(R_curves [m])  "<R_curve>"
  Car_Rx_1_BusSelector/2 -> INTERPRETATION ALGORITHM TrafficSign Intelligence/11(notifications [-])  "<permission>"
  Car_Rx_1_Demux/5(Velocity_[m/s]) -> Terminator_1/1
  Car_Rx_1_Demux/6(Acceleration_[m/s2]) -> Terminator_2/1
  Car_Rx_1_Demux/8(warning_signal_id) -> Terminator_3/1
  Car_Rx_1_Demux/9(maneuvre_id) -> Terminator_4/1
  Car_Rx_1_Demux/10(vehicle_type) -> Terminator_5/1
  Car_Rx_1_Demux/11(brakingcapacity) -> Terminator_6/1
  Car_Rx_1_Demux/12(vehicle_size) -> Terminator_7/1
  SELF_Demux/3(z [m]) -> Terminator_8/1
  SELF_Demux/4(rotx [deg]) -> Terminator_9/1
  SELF_Demux/5(roty [deg]) -> Terminator_10/1
  SELF_Demux/6(rotz [deg]) -> Terminator_11/1
  SELF_Demux/7(GPS lat [deg]) -> Terminator_12/1
  SELF_Demux/8(GPS long [deg]) -> Terminator_13/1
  SELF_Demux/9(GPS alt [m]) -> Terminator_14/1
  SELF_Demux/12(yaw rate [deg/s]) -> Terminator_15/1
  Ground_1/1 -> VISULightSource_Mux/1(IndicatorLightsLeft)
  Ground_2/1 -> VISULightSource_Mux/2(IndicatorLightsRight)
  Ground_3/1 -> VISULightSource_Mux/4(FogLightRearRight)
  Ground_4/1 -> VISULightSource_Mux/5(FogLightFrontLeft)
  Ground_5/1 -> VISULightSource_Mux/6(FogLightFrontRight)
  Ground_6/1 -> VISULightSource_Mux/7(MainLightRearLeft)
  Ground_7/1 -> VISULightSource_Mux/8(MainLightRearRight)
  Ground_8/1 -> VISULightSource_Mux/9(MainLightFrontLeft)
  Ground_9/1 -> VISULightSource_Mux/10(MainLightFrontRight)
  Ground_10/1 -> VISUWheels_Mux/2(Steer Angle Rear [deg])

== system Demo_IntelligentTrafficSigns_cs/CameraTrolley ==
  SELF_CameraTrolley : Inport  Tag=SELFInport
  Dynamics_Empty : Subsystem  Tag=Dynamics_Empty
      mask Display: port_label('output', 1, 'State');text(0,0,' Empty\n')
  SELF_Demux : Subsystem  Tag=DemuxSELF
      settings: DeleteFcn=PreScanBlockDeleteFcn;
      mask Type: PreScan Demux Block:
      mask Display:
      | port_label('output',1,'x [m]')
      | port_label('output',2,'y [m]')
      | port_label('output',3,'z [m]','texmode','on')
      | port_label('output',4,'Rotx [deg]','texmode','on')
      | port_label('output',5,'Roty [deg]','texmode','on')
      | port_label('output',6,'Rotz [deg]','texmode','on')
      | port_label('output',7,'GPS lat [deg]','texmode','on')
      | port_label('output',8,'GPS long [deg]','texmode','on')
      | port_label('output',9,'GPS alt [m]','texmode','on')
      | port_label('output',10,'Velocity [m/s]')
      | port_label('output',11,'Heading [deg]')
      | port_label('output',12,'Yaw rate [deg/s]')
  Terminator : Terminator
  Terminator_{1..12} (12 alike) : Terminator
  transmits only position : Gain  Gain=[1 1 1 0 0 0 0 0 1]
  STATE_CameraTrolley : Outport  Tag=StateOutport
  wires:
  [State_BMW] -> transmits only position/1
  SELF_CameraTrolley/1 -> SELF_Demux/1(SELF)
  Dynamics_Empty/1(State) -> Terminator/1
  transmits only position/1 -> STATE_CameraTrolley/1
  SELF_Demux/1(x [m]) -> Terminator_1/1
  SELF_Demux/2(y [m]) -> Terminator_2/1
  SELF_Demux/3(z [m]) -> Terminator_3/1
  SELF_Demux/4(rotx [deg]) -> Terminator_4/1
  SELF_Demux/5(roty [deg]) -> Terminator_5/1
  SELF_Demux/6(rotz [deg]) -> Terminator_6/1
  SELF_Demux/7(GPS lat [deg]) -> Terminator_7/1
  SELF_Demux/8(GPS long [deg]) -> Terminator_8/1
  SELF_Demux/9(GPS alt [m]) -> Terminator_9/1
  SELF_Demux/10(velocity [m/s]) -> Terminator_10/1
  SELF_Demux/11(heading [deg]) -> Terminator_11/1
  SELF_Demux/12(yaw rate [deg/s]) -> Terminator_12/1

== system Demo_IntelligentTrafficSigns_cs/Car_Rx_1 ==
  Selector : Selector  IndexMode=Zero-based  IndexOptions=Starting index (dialog)  Indices=PortIndex  OutputSizes=PortWidth(1)
  U : Outport
  wires:
  Selector/1 -> U/1
  [SimCoreSensors] -> Selector/1

== system Demo_IntelligentTrafficSigns_cs/GetSensors ==
  dummy : Inport
  GetSensors : M-S-Function  FunctionName=GetSensorsBlock  Parameters=TimeStep NrOfSensors
  tag scope: SimCoreSensors=global
  wires:
  GetSensors/1 -> [SimCoreSensors]
  dummy/1 -> GetSensors/1

== system Demo_IntelligentTrafficSigns_cs/SELF_BMW_X5_1 ==
  Selector : Selector  IndexMode=Zero-based  IndexOptions=Starting index (dialog)  Indices=PortIndex  OutputSizes=PortWidth(1)
  U : Outport
  wires:
  Selector/1 -> U/1
  [SimCoreSensors] -> Selector/1

== system Demo_IntelligentTrafficSigns_cs/SELF_CameraTrolley ==
  Selector : Selector  IndexMode=Zero-based  IndexOptions=Starting index (dialog)  Indices=PortIndex  OutputSizes=PortWidth(1)
  U : Outport
  wires:
  Selector/1 -> U/1
  [SimCoreSensors] -> Selector/1
